simulink model "BaseEngineController_A02_bak"  (Simulink 7.7)
settings: Solver=FixedStepDiscrete  StartTime=0.0  StopTime=10.0  RelTol=1e-3
PreLoadFcn:
  | try, eval([bdroot '_setup']); catch, eval('model_setup', ''); end
InitFcn:
  | try, eval([bdroot '_setup']); catch, eval('model_setup', ''); end
undefined symbols (not defined in the file or in its folder): ECU555, High, motohawk_build_version, motohawk_get_faults, motohawk_trunc_name, motohawk_vardec_config_group, motohawk_vardec_path, True
libraries: MotoHawk_lib x1145, AutoDoc_lib x786, motohawk_control_primitives_lib x114, motohawk_engine_lib x19, motohawk_annotations_lib x12, simulink x11, simulink_extras x1
(part 5 of 23)

== system BaseEngineController_A02_bak/Foreground/Control/Controller/Stall ==
  Enable : EnablePort  StatesWhenEnabling=reset
  Bus Creator2 : BusCreator  Inputs=11
  Closed Throttle : Constant  Value=0
  Documentation_Calibrations_Probes_Block : AutoDoc_lib/Documentation_Calibrations_Probes_Block
  Documentation_Left_Border : AutoDoc_lib/Documentation_Left_Border  layout=Portrait
  Documentation_Right_Border : AutoDoc_lib/Documentation_Right_Border  layout=Portrait
  Documentation_Title_Block : AutoDoc_lib/Documentation_Title_Block
  EGR : Constant  Value=0  OutDataTypeStr=double
  Fuel Pump at Startup : Subsystem
  No Fuel : Constant  Value=0
  No Mult : Constant  Value=0  OutDataTypeStr=double
  No Spark : Constant  Value=0
  No Spark Timing : Constant  Value=0
  No Start EOI : Constant  Value=0
  No Start Fuel Timing : Constant  Value=0
  O2 Heater : Constant  OutDataTypeStr=boolean
  Oil Pump at Startup : Subsystem
  Zero-Order Hold{1..11} (11 alike) : ZeroOrderHold  SampleTime=-1
  motohawk_replicate : MotoHawk_lib/Extra Development Blocks/motohawk_replicate  output_width=-1
  motohawk_replicate2 : MotoHawk_lib/Extra Development Blocks/motohawk_replicate  output_width=-1
  Actuators : Outport
  wires:
  Zero-Order Hold6/1 -> Bus Creator2/1  "FPC"
  No Spark/1 -> Zero-Order Hold4/1
  No Spark Timing/1 -> motohawk_replicate2/1
  No Fuel/1 -> motohawk_replicate/1
  Zero-Order Hold8/1 -> Bus Creator2/2  "SOI"
  No Start Fuel Timing/1 -> Zero-Order Hold8/1
  Bus Creator2/1 -> Actuators/1
  Closed Throttle/1 -> Zero-Order Hold9/1
  Zero-Order Hold4/1 -> Bus Creator2/4  "SparkEnergy"
  Zero-Order Hold3/1 -> Bus Creator2/5  "SparkAdv"
  Zero-Order Hold9/1 -> Bus Creator2/6  "Throttle"
  Zero-Order Hold7/1 -> Bus Creator2/7  "FUELP"
  motohawk_replicate/1 -> Zero-Order Hold6/1
  Zero-Order Hold5/1 -> Bus Creator2/3  "EOI"
  No Start EOI/1 -> Zero-Order Hold5/1
  motohawk_replicate2/1 -> Zero-Order Hold3/1
  No Mult/1 -> Zero-Order Hold10/1
  Zero-Order Hold10/1 -> Bus Creator2/8  "FuelMult"
  Fuel Pump at Startup/1(FUELP [bool]) -> Zero-Order Hold7/1
  EGR/1 -> Zero-Order Hold1/1
  Zero-Order Hold1/1 -> Bus Creator2/9  "EGR"
  Oil Pump at Startup/1(OILP [bool]) -> Zero-Order Hold2/1
  Zero-Order Hold2/1 -> Bus Creator2/10  "OILP"
  O2 Heater/1 -> Zero-Order Hold11/1
  Zero-Order Hold11/1 -> Bus Creator2/11  "O2 Heater"

== system BaseEngineController_A02_bak/Foreground/Control/Diagnostics/Engine Overheat Fault Logic ==
  Sensors : Inport
  Bus Selector : BusSelector  OutputSignals=Raw.NonLinearSensors.ECT
  Documentation_Calibrations_Probes_Block : AutoDoc_lib/Documentation_Calibrations_Probes_Block
  Documentation_Left_Border : AutoDoc_lib/Documentation_Left_Border  layout=Portrait
  Documentation_Right_Border : AutoDoc_lib/Documentation_Right_Border  layout=Portrait
  Documentation_Title_Block : AutoDoc_lib/Documentation_Title_Block
  Logical Operator1 : Logic  Operator=OR  AllPortsSameDT=off  OutDataTypeStr=boolean
  Logical Operator2 : Logic  Inputs=3  AllPortsSameDT=off  OutDataTypeStr=boolean
  Logical Operator3 : Logic  Operator=NOT  Inputs=3  AllPortsSameDT=off  OutDataTypeStr=boolean
  Logical Operator4 : Logic  Inputs=3  AllPortsSameDT=off  OutDataTypeStr=boolean
  Relational Operator1 : RelationalOperator  OutDataTypeStr=boolean
  Relational Operator2 : RelationalOperator  OutDataTypeStr=boolean
  Relational Operator3 : RelationalOperator  OutDataTypeStr=boolean
  Run State : motohawk_control_primitives_lib/CompareTo  SystemSampleTime=-1  op===  val=3
  motohawk_calibration1 : MotoHawk_lib/Calibration & Probing Blocks/motohawk_calibration  nam='TempOverheat_Warn'  val=95  storage=Calibration  show_more=on  name_source=Use Parameter  data_type=double  read_level=1  write_level=1  ovr_cals=on  view_as=Number  enum_struct={'Disabled', 'Enabled'}  vector_style=Wide Row  use_help_units=on  help='ECT limit above which warning light comes on'  units='degC'  use_header_enum=off  row_header_enum={}  col_header_enum={}  use_min_max=off  min_val=-inf  max_val=inf  use_precision=off  precision=''  gain=1  offset=0  exp=1  use_mototune_group=on  mototune_group=motohawk_vardec_path(gcb)
  motohawk_calibration2 : MotoHawk_lib/Calibration & Probing Blocks/motohawk_calibration  nam='TempOverheat_Fault'  val=100  storage=Calibration  show_more=on  name_source=Use Parameter  data_type=double  read_level=1  write_level=1  ovr_cals=on  view_as=Number  enum_struct={'Disabled', 'Enabled'}  vector_style=Wide Row  use_help_units=on  help='ECT limit above which fault is set.'  units='degC'  use_header_enum=off  row_header_enum={}  col_header_enum={}  use_min_max=off  min_val=-inf  max_val=inf  use_precision=off  precision=''  gain=1  offset=0  exp=1  use_mototune_group=on  mototune_group=motohawk_vardec_path(gcb)
  motohawk_calibration3 : MotoHawk_lib/Calibration & Probing Blocks/motohawk_calibration  nam='TempOverheatSevere_Fault'  val=110  storage=Calibration  show_more=on  name_source=Use Parameter  data_type=double  read_level=1  write_level=1  ovr_cals=on  view_as=Number  enum_struct={'Disabled', 'Enabled'}  vector_style=Wide Row  use_help_units=on  help='ECT limit above which severe overheat fault is set.'  units='degC'  use_header_enum=off  row_header_enum={}  col_header_enum={}  use_min_max=off  min_val=-inf  max_val=inf  use_precision=off  precision=''  gain=1  offset=0  exp=1  use_mototune_group=on  mototune_group=motohawk_vardec_path(gcb)
  motohawk_data_read : MotoHawk_lib/Data Storage Blocks/motohawk_data_read  source=Lookup By Name  structure=Scalar  operation=Read entire data structure at once  sz_vec=4  sz_row=2  sz_col=3  typ=uint8  nam='EngineState'  checkNull=on  struct_inst_name='Struct1'  struct_nam='MyStruct'
  motohawk_fault_def1 : MotoHawk_lib/Fault Management Blocks/motohawk_fault_def  nam='ECTOverTempFault'  mode=Enabled  id=[]  x=25  y=50  input_suspected=on  indeterminate=off  increment_xy=on  downsample_count=1  ovr_cals=on  ovr_actions=on  show_actions=off  action1_name=''  action1_cond=(None)  action2_name=''  action2_cond=(None)  action3_name=''  action3_cond=(None)  action4_name=''  action4_cond=(None)
  motohawk_fault_def2 : MotoHawk_lib/Fault Management Blocks/motohawk_fault_def  nam='ECTSevereOverTempFault'  mode=Enabled  id=[]  x=25  y=50  input_suspected=on  indeterminate=off  increment_xy=on  downsample_count=1  ovr_cals=on  ovr_actions=on  show_actions=off  action1_name=''  action1_cond=(None)  action2_name=''  action2_cond=(None)  action3_name=''  action3_cond=(None)  action4_name=''  action4_cond=(None)
  motohawk_fault_status : MotoHawk_lib/Fault Management Blocks/motohawk_fault_status  source=Lookup By Name  nam='ECTRangeHigh'  status=Active
  motohawk_fault_status1 : MotoHawk_lib/Fault Management Blocks/motohawk_fault_status  source=Lookup By Name  nam='ECTRangeLow'  status=Active
  Overheat_Warning : Outport
  wires:
  motohawk_data_read/1 -> Run State/1
  Bus Selector/1 -> Relational Operator3/1, Relational Operator1/1, Relational Operator2/1  "<ECT>"
  motohawk_calibration2/1 -> Relational Operator3/2
  Sensors/1 -> Bus Selector/1
  Relational Operator3/1 -> Logical Operator2/3
  Logical Operator2/1 -> motohawk_fault_def1/1
  Run State/1 -> Logical Operator2/2, Logical Operator4/2
  motohawk_fault_status/1 -> Logical Operator1/1
  motohawk_fault_status1/1 -> Logical Operator1/2
  Logical Operator1/1 -> Logical Operator3/1
  Logical Operator3/1 -> Logical Operator2/1, Logical Operator4/1
  motohawk_calibration1/1 -> Relational Operator1/2
  Relational Operator1/1 -> Overheat_Warning/1
  motohawk_calibration3/1 -> Relational Operator2/2
  Relational Operator2/1 -> Logical Operator4/3
  Logical Operator4/1 -> motohawk_fault_def2/1

== system BaseEngineController_A02_bak/Foreground/Control/Diagnostics/Fault Codes and States ==
  IC : Inport
  Add : Sum  Inputs=+-  OutDataTypeStr=Inherit: Same as first input
  Constant : Constant  Value=uint8(1)
  Constant1 : Constant  Value=length(motohawk_get_faults(bdroot))
  Data Type Conversion : DataTypeConversion  SaturateOnIntegerOverflow=on  RndMeth=Zero
  Data Type Conversion1 : DataTypeConversion  SaturateOnIntegerOverflow=on  RndMeth=Zero
  Documentation_Calibrations_Probes_Block : AutoDoc_lib/Documentation_Calibrations_Probes_Block
  Documentation_Left_Border : AutoDoc_lib/Documentation_Left_Border  layout=Portrait
  Documentation_Right_Border : AutoDoc_lib/Documentation_Right_Border  layout=Portrait
  Documentation_Title_Block : AutoDoc_lib/Documentation_Title_Block
  Relational Operator : RelationalOperator  Operator=<  OutDataTypeStr=boolean
  Terminator : Terminator
  While Iterator : WhileIterator  MaxIters=-1  ResetStates=reset  ShowIterationPort=on  OutputDataType=int8
  motohawk_data_def : MotoHawk_lib/Data Storage Blocks/motohawk_data_def  nam='FltCodes'  container_nam=''  data=zeros(length(motohawk_get_faults(bdroot)),1)  typ=uint16  struct_nam='MyStruct'  storage=Volatile  outputRef=off  use_vardec=off  window=Display  read_level=1  write_level=(Read Only)  ovr_cals=on  view_as=Number  enum_struct={'Disabled', 'Enabled'}  vector_style=Tall Column  use_help_units=off  help=''  units=''  use_header_enum=off  row_header_enum=''  col_header_enum=''  use_min_max=off  min_val=-inf  max_val=inf  use_precision=off  precision=''  gain=1  offset=0  exp=1  use_mototune_group=on  mototune_group='TESTING'  eval_name=FltCodes  data_stores=motohawk_sfun_data_def_data_stores  struct_instances=motohawk_sfun_data_def_struct_instances  vardecs=motohawk_sfun_data_def_vardecs
  motohawk_data_def1 : MotoHawk_lib/Data Storage Blocks/motohawk_data_def  nam='FltActvStatus'  container_nam=''  data=zeros(length(motohawk_get_faults(bdroot)),1)  typ=boolean  struct_nam='MyStruct'  storage=Volatile  outputRef=off  use_vardec=off  window=Display  read_level=1  write_level=(Read Only)  ovr_cals=on  view_as=Number  enum_struct={'Disabled', 'Enabled'}  vector_style=Tall Column  use_help_units=off  help=''  units=''  use_header_enum=off  row_header_enum=''  col_header_enum=''  use_min_max=off  min_val=-inf  max_val=inf  use_precision=off  precision=''  gain=1  offset=0  exp=1  use_mototune_group=on  mototune_group='TESTING'  eval_name=FltActvStatus  data_stores=motohawk_sfun_data_def_data_stores  struct_instances=motohawk_sfun_data_def_struct_instances  vardecs=motohawk_sfun_data_def_vardecs
  motohawk_data_write : MotoHawk_lib/Data Storage Blocks/motohawk_data_write  source=Lookup By Name  structure=Vector  operation=Write scalar into location by index  sz_vec=-1  sz_row=3  sz_col=2  typ=uint16  nam='FltCodes'  checkNull=on  write_read_only=off  struct_inst_name='Struct1'  struct_name='MyStruct'
  motohawk_data_write1 : MotoHawk_lib/Data Storage Blocks/motohawk_data_write  source=Lookup By Name  structure=Vector  operation=Write scalar into location by index  sz_vec=-1  sz_row=3  sz_col=2  typ=boolean  nam='FltActvStatus'  checkNull=on  write_read_only=off  struct_inst_name='Struct1'  struct_name='MyStruct'
  motohawk_fault_iterator : MotoHawk_lib/Fault Management Blocks/motohawk_fault_iterator  criteria=All Faults  loop=off
  motohawk_fault_properties : MotoHawk_lib/Fault Management Blocks/motohawk_fault_properties  source=Input Reference Signal  nam='Fault1'  use_id=on  use_mode=off  use_xlim=off  use_ylim=off  use_x=off  use_y=off
  motohawk_fault_status : MotoHawk_lib/Fault Management Blocks/motohawk_fault_status  source=Input Reference Signal  nam='Fault1'  status=Active
  motohawk_prev_fault_T : MotoHawk_lib/Fault Management Blocks/motohawk_prev_fault_T
  wires:
  Constant/1 -> Add/2
  Data Type Conversion/1 -> Add/1, Relational Operator/1
  Relational Operator/1 -> While Iterator/1
  While Iterator/1 -> Data Type Conversion/1
  Constant1/1 -> Relational Operator/2
  IC/1 -> While Iterator/2
  motohawk_fault_iterator/2 -> motohawk_fault_properties/1, motohawk_prev_fault_T/1, motohawk_fault_status/1
  motohawk_fault_iterator/1 -> Terminator/1
  motohawk_fault_properties/1 -> Data Type Conversion1/1
  Add/1 -> motohawk_data_write/2, motohawk_data_write1/2
  motohawk_fault_status/1 -> motohawk_data_write1/1
  Data Type Conversion1/1 -> motohawk_data_write/1
  motohawk_prev_fault_T/1 -> motohawk_fault_iterator/1

== system BaseEngineController_A02_bak/Foreground/Control/Diagnostics/Freeze Frame Trigger ==
  Active Fault Array : MotoHawk_lib/Fault Management Blocks/motohawk_fault_statuses  nam=motohawk_get_faults(bdroot)  status=Active
  Add : Sum  Inputs=+-  OutDataTypeStr=int8
  Data Type Conversion1 : DataTypeConversion  OutDataTypeStr=boolean  SaturateOnIntegerOverflow=on  RndMeth=Zero
  Documentation_Calibrations_Probes_Block : AutoDoc_lib/Documentation_Calibrations_Probes_Block
  Documentation_Left_Border : AutoDoc_lib/Documentation_Left_Border  layout=Portrait
  Documentation_Right_Border : AutoDoc_lib/Documentation_Right_Border  layout=Portrait
  Documentation_Title_Block : AutoDoc_lib/Documentation_Title_Block
  Freeze-Frame Trigger : Stateflow chart  TreatAsAtomicUnit=on
  Ground1 : Ground
  Initialized at 1 : UnitDelay  X0=1  SampleTime=-1
  Multiport Switch : MultiPortSwitch  DataPortOrder=Zero-based contiguous  Inputs=2  zeroidx=on  InputSameDT=on
  Saturation : Saturate  UpperLimit=1  LowerLimit=0
  Unit Delay2 : UnitDelay  SampleTime=-1
  Width1 : Width  DataType=uint8
  Width2 : Width  DataType=uint8
  motohawk_data_def1 : MotoHawk_lib/Data Storage Blocks/motohawk_data_def  nam='FFIDs'  container_nam=''  data=zeros(6,1)  typ=uint8  struct_nam='MyStruct'  storage=NonVolatile  outputRef=off  use_vardec=off  window=Display  read_level=1  write_level=(Read Only)  ovr_cals=on  view_as=Number  enum_struct={'Disabled', 'Enabled'}  vector_style=Tall Column  use_help_units=off  help=''  units=''  use_header_enum=off  row_header_enum={}  col_header_enum={}  use_min_max=off  min_val=-inf  max_val=inf  use_precision=off  precision=''  gain=1  offset=0  exp=1  use_mototune_group=on  mototune_group='TESTING'  eval_name=FFIDs  data_stores=motohawk_sfun_data_def_data_stores  struct_instances=motohawk_sfun_data_def_struct_instances  vardecs=motohawk_sfun_data_def_vardecs
  motohawk_data_def2 : MotoHawk_lib/Data Storage Blocks/motohawk_data_def  nam='FltOrder'  container_nam=''  data=zeros(length(motohawk_get_faults(bdroot)),1)  typ=uint16  struct_nam='MyStruct'  storage=NonVolatile  outputRef=off  use_vardec=off  window=Display  read_level=1  write_level=(Read Only)  ovr_cals=on  view_as=Number  enum_struct={'Disabled', 'Enabled'}  vector_style=Tall Column  use_help_units=off  help=''  units=''  use_header_enum=off  row_header_enum={}  col_header_enum={}  use_min_max=off  min_val=-inf  max_val=inf  use_precision=off  precision=''  gain=1  offset=0  exp=1  use_mototune_group=on  mototune_group='TESTING'  eval_name=FltOrder  data_stores=motohawk_sfun_data_def_data_stores  struct_instances=motohawk_sfun_data_def_struct_instances  vardecs=motohawk_sfun_data_def_vardecs
  motohawk_data_def3 : MotoHawk_lib/Data Storage Blocks/motohawk_data_def  nam='FltOrderMax'  container_nam=''  data=0  typ=uint16  struct_nam='MyStruct'  storage=NonVolatile  outputRef=off  use_vardec=off  window=Display  read_level=1  write_level=(Read Only)  ovr_cals=on  view_as=Number  enum_struct={'Disabled', 'Enabled'}  vector_style=Tall Column  use_help_units=off  help=''  units=''  use_header_enum=off  row_header_enum={}  col_header_enum={}  use_min_max=off  min_val=-inf  max_val=inf  use_precision=off  precision=''  gain=1  offset=0  exp=1  use_mototune_group=off  mototune_group='TESTING'  eval_name=FltOrderMax  data_stores=motohawk_sfun_data_def_data_stores  struct_instances=motohawk_sfun_data_def_struct_instances  vardecs=motohawk_sfun_data_def_vardecs
  motohawk_data_read1 : MotoHawk_lib/Data Storage Blocks/motohawk_data_read  source=Lookup By Name  structure=Vector  operation=Read entire data structure at once  sz_vec=length(motohawk_get_faults(bdroot))  sz_row=2  sz_col=3  typ=uint16  nam='FltOrder'  checkNull=on  struct_inst_name='Struct1'  struct_nam='MyStruct'
  motohawk_data_read2 : MotoHawk_lib/Data Storage Blocks/motohawk_data_read  source=Lookup By Name  structure=Scalar  operation=Read entire data structure at once  sz_vec=10  sz_row=2  sz_col=3  typ=uint16  nam='FltOrderMax'  checkNull=on  struct_inst_name='Struct1'  struct_nam='MyStruct'
  motohawk_data_read3 : MotoHawk_lib/Data Storage Blocks/motohawk_data_read  source=Lookup By Name  structure=Vector  operation=Read entire data structure at once  sz_vec=6  sz_row=2  sz_col=3  typ=uint8  nam='FFIDs'  checkNull=on  struct_inst_name='Struct1'  struct_nam='MyStruct'
  motohawk_data_write1 : MotoHawk_lib/Data Storage Blocks/motohawk_data_write  source=Lookup By Name  structure=Vector  operation=Write entire data structure at once  sz_vec=6  sz_row=10  sz_col=1  typ=uint8  nam='FFIDs'  checkNull=on  write_read_only=off  struct_inst_name='Struct1'  struct_name='MyStruct'
  motohawk_data_write2 : MotoHawk_lib/Data Storage Blocks/motohawk_data_write  source=Lookup By Name  structure=Vector  operation=Write entire data structure at once  sz_vec=length(motohawk_get_faults(bdroot))  sz_row=10  sz_col=1  typ=uint16  nam='FltOrder'  checkNull=on  write_read_only=off  struct_inst_name='Struct1'  struct_name='MyStruct'
  motohawk_data_write3 : MotoHawk_lib/Data Storage Blocks/motohawk_data_write  source=Lookup By Name  structure=Scalar  operation=Write entire data structure at once  sz_vec=10  sz_row=10  sz_col=1  typ=uint16  nam='FltOrderMax'  checkNull=on  write_read_only=off  struct_inst_name='Struct1'  struct_name='MyStruct'
  zeros vector : Constant  Value=zeros(length(motohawk_get_faults(bdroot)),1)  OutDataTypeStr=int8
  zeros vector1 : Constant  Value=[0 0 0 0 0 0]  OutDataTypeStr=boolean
  IDs : Outport
  Trigger : Outport  Port=2
  wires:
  Unit Delay2/1 -> Add/2
  Add/1 -> Saturation/1
  Saturation/1 -> Multiport Switch/2
  Freeze-Frame Trigger/1(FFIDs) -> motohawk_data_write1/1, IDs/1
  motohawk_data_read3/1 -> Freeze-Frame Trigger/3(FFIDsIn), Width1/1
  Width1/1 -> Freeze-Frame Trigger/4(NumFF)
  Freeze-Frame Trigger/3(FltOrder) -> motohawk_data_write2/1
  motohawk_data_read1/1 -> Freeze-Frame Trigger/5(FltOrderIn), Width2/1
  Freeze-Frame Trigger/4(FltOrderMax) -> motohawk_data_write3/1
  motohawk_data_read2/1 -> Freeze-Frame Trigger/7(FltOrderMaxIn)
  Width2/1 -> Freeze-Frame Trigger/6(NumFlts)
  Freeze-Frame Trigger/2(FFTrigg) -> Trigger/1
  zeros vector/1 -> Multiport Switch/3
  Active Fault Array/1 -> Add/1, Unit Delay2/1
  Multiport Switch/1 -> Freeze-Frame Trigger/1(ActvFlts)
  Initialized at 1/1 -> Data Type Conversion1/1
  Ground1/1 -> Initialized at 1/1
  Data Type Conversion1/1 -> Multiport Switch/1
  zeros vector1/1 -> Freeze-Frame Trigger/2(FFTriggZeros)

== system BaseEngineController_A02_bak/Foreground/Control/Diagnostics/Freeze Frames ==
  Data : Inport
  IDs : Inport  Port=2
  Trigger : Inport  Port=3
  Clear FFIDs and FltOrder : Subsystem  TreatAsAtomicUnit=on
  Demux : Demux  Outputs=6
  Demux1 : Demux  Outputs=6
  Documentation_Calibrations_Probes_Block : AutoDoc_lib/Documentation_Calibrations_Probes_Block
  Documentation_Left_Border : AutoDoc_lib/Documentation_Left_Border  layout=Portrait
  Documentation_Right_Border : AutoDoc_lib/Documentation_Right_Border  layout=Portrait
  Documentation_Title_Block : AutoDoc_lib/Documentation_Title_Block
  Freeze Frame Data Storage : Subsystem  TreatAsAtomicUnit=on  mask{FFnum=1}
      mask prompts: FFnum "Freeze Frame Number"
      mask init:
      | % Color
      | r = 220;
      | g = 211;
      | b = 254;
      | color = strcat('[', num2str(r/255), ',', num2str(g/255), ',', num2str(b/255), ']');
      | motohawk_set_param(gcb, 'BackgroundColor', ...
      |     color);
      | 
      | % Size
      | p = get_param(gcb, 'Position');
      | ht = 52;
      | wd = 290;
      | motohawk_set_param(gcb, 'Position', [p(1) p(2) (p(1) + wd) (p(2) + ht)]);
      | 
      | %Label
      | lbl = ['Freeze-Frame Number: ' num2str(FFnum)];
      mask Display: disp(lbl)
  Freeze Frame Data Storage1 : Subsystem  TreatAsAtomicUnit=on  mask{FFnum=2}
      mask prompts: FFnum "Freeze Frame Number"
      mask init: same as BaseEngineController_A02_bak/Foreground/Control/Diagnostics/Freeze Frames/Freeze Frame Data Storage
      mask Display: disp(lbl)
  Freeze Frame Data Storage2 : Subsystem  TreatAsAtomicUnit=on  mask{FFnum=3}
      mask prompts: FFnum "Freeze Frame Number"
      mask init: same as BaseEngineController_A02_bak/Foreground/Control/Diagnostics/Freeze Frames/Freeze Frame Data Storage
      mask Display: disp(lbl)
  Freeze Frame Data Storage3 : Subsystem  TreatAsAtomicUnit=on  mask{FFnum=4}
      mask prompts: FFnum "Freeze Frame Number"
      mask init: same as BaseEngineController_A02_bak/Foreground/Control/Diagnostics/Freeze Frames/Freeze Frame Data Storage
      mask Display: disp(lbl)
  Freeze Frame Data Storage4 : Subsystem  TreatAsAtomicUnit=on  mask{FFnum=5}
      mask prompts: FFnum "Freeze Frame Number"
      mask init: same as BaseEngineController_A02_bak/Foreground/Control/Diagnostics/Freeze Frames/Freeze Frame Data Storage
      mask Display: disp(lbl)
  Freeze Frame Data Storage5 : Subsystem  TreatAsAtomicUnit=on  mask{FFnum=6}
      mask prompts: FFnum "Freeze Frame Number"
      mask init: same as BaseEngineController_A02_bak/Foreground/Control/Diagnostics/Freeze Frames/Freeze Frame Data Storage
      mask Display: disp(lbl)
  Logical Operator : Logic  Operator=OR  OutDataTypeStr=boolean
  motohawk_calibration : MotoHawk_lib/Calibration & Probing Blocks/motohawk_calibration  nam='ClearFreezeFrames'  val=0  storage=Display  show_more=on  name_source=Use Parameter  data_type=boolean  read_level=1  write_level=1  ovr_cals=on  view_as=Enumeration  enum_struct={'No Clear', 'Clear'}  vector_style=Wide Row  use_help_units=on  help='Manual clearing of freeze-frame data'  units=''  use_header_enum=off  row_header_enum={}  col_header_enum={}  use_min_max=off  min_val=-inf  max_val=inf  use_precision=off  precision=''  gain=1  offset=0  exp=1  use_mototune_group=on  mototune_group=motohawk_vardec_path(gcb)
  motohawk_data_def : MotoHawk_lib/Data Storage Blocks/motohawk_data_def  nam='MyData'  container_nam='FreezeFramesContainer'  data={'FF1', 'FF2','FF3','FF4','FF5','FF6'}  typ=struct container  struct_nam='FreezeFrames'  storage=Volatile  outputRef=off  use_vardec=on  window=Display  read_level=1  write_level=(Read Only)  ovr_cals=on  view_as=Number  enum_struct={'Disabled', 'Enabled'}  vector_style=Wide Row  use_help_units=off  help=''  units=''  use_header_enum=off  row_header_enum=''  col_header_enum=''  use_min_max=off  min_val=-inf  max_val=inf  use_precision=off  precision=''  gain=1  offset=0  exp=1  use_mototune_group=on  mototune_group='Diagnostics & Protection | Faults | Freeze Frame'  eval_name=MyData  data_stores=motohawk_sfun_data_def_data_stores  struct_instances=motohawk_sfun_data_def_struct_instances  vardecs=motohawk_sfun_data_def_vardecs
  motohawk_struct_decl : MotoHawk_lib/Data Storage Blocks/motohawk_struct_decl  struct_nam='FreezeFrames'  data=struct('Name', int32(0), 'Code', int32(0), 'Var1', int32(0), 'Var2', int32(0), 'Var3', int32(0), 'Var4', int32(0), 'Var5', int32(0), 'Var6', int32(0), 'Var7', int32(0), 'Var8', int32(0), 'Var9', int32(0), 'Var10', int32(0))  enum_defs={['None'; motohawk_get_faults(bdroot)]}
  wires:
  Logical Operator/1 -> Demux1/1
  IDs/1 -> Demux/1
  Demux/1 -> Freeze Frame Data Storage/1(FaultID)
  Demux/2 -> Freeze Frame Data Storage1/1(FaultID)
  Demux/3 -> Freeze Frame Data Storage2/1(FaultID)
  Demux/4 -> Freeze Frame Data Storage3/1(FaultID)
  Demux/5 -> Freeze Frame Data Storage4/1(FaultID)
  Demux/6 -> Freeze Frame Data Storage5/1(FaultID)
  Data/1 -> Freeze Frame Data Storage4/2(Data), Freeze Frame Data Storage5/2(Data), Freeze Frame Data Storage3/2(Data), Freeze Frame Data Storage2/2(Data), Freeze Frame Data Storage1/2(Data), Freeze Frame Data Storage/2(Data)
  Demux1/1 -> Freeze Frame Data Storage/Trigger
  Demux1/2 -> Freeze Frame Data Storage1/Trigger
  Demux1/3 -> Freeze Frame Data Storage2/Trigger
  Demux1/4 -> Freeze Frame Data Storage3/Trigger
  Demux1/5 -> Freeze Frame Data Storage4/Trigger
  Demux1/6 -> Freeze Frame Data Storage5/Trigger
  Trigger/1 -> Logical Operator/2
  motohawk_calibration/1 -> Logical Operator/1, Clear FFIDs and FltOrder/Trigger

== system BaseEngineController_A02_bak/Foreground/Control/Diagnostics/Fuel Economy ==
  Sensors : Inport
  Controller : Inport  Port=2
  Bus Selector : BusSelector  OutputSignals=Raw.CAN Inputs.112.Vehicle Speed
  Bus Selector1 : BusSelector  OutputSignals=FPC
  Bus Selector2 : BusSelector  OutputSignals=Raw.RPM
  Bus Selector3 : BusSelector  OutputSignals=Raw.LinearSensors.FuelSense
  Calculate Fuel Density : Subsystem
  Constant1 : Constant
  Constant2 : Constant
  Data Type Conversion : DataTypeConversion  SaturateOnIntegerOverflow=on  RndMeth=Zero
  Default (if no flow) : Switch  Threshold=0.5  ZeroCross=off  SaturateOnIntegerOverflow=on  InputSameDT=on
  Default value : Constant  Value=0
  Documentation_Calibrations_Probes_Block : AutoDoc_lib/Documentation_Calibrations_Probes_Block
  Documentation_Left_Border : AutoDoc_lib/Documentation_Left_Border  layout=Portrait
  Documentation_Right_Border : AutoDoc_lib/Documentation_Right_Border  layout=Portrait
  Documentation_Title_Block : AutoDoc_lib/Documentation_Title_Block
  First Order Low Pass (Tunable) : Subsystem  AncestorBlock=motohawk_control_primitives_lib/First Order Low Pass (Tunable)  mask(First-Order Low-Pass Filter (Tunable)){nam='FuelEconomy_Filter', samp_time=-1, group=motohawk_vardec_path(gcb)}
      mask prompts: nam "Name"; samp_time "Sample Time"; group "MotoTune Group String"
      mask help:
      | Discrete first-order low-pass filter, with calibratable time-constant.
      |
      | If 'Sample Time' is -1, then extra code and RAM will be generated to dynamically calculate dt.
      mask Display:
      | patch([0 0 1 1], [0 0.075 0.075 0], [230/255 0/255 0/255])
      | patch([0 0 1 1], [0.025 0.05 0.05 0.025], [150/255 0/255 0/255])
      | patch([0 0 1 1], [0 0.025 0.025 0], [0/255 0/255 0/255])
      | 
      | disp('1st-Order\nLow Pass');
  Mass flow to Volume flow mg/hr to ml/hr : Product  Inputs=*/  SaturateOnIntegerOverflow=on  InputSameDT=on  OutDataTypeStr=Inherit: Same as first input
  Min fuel flow  for calc : Constant  Value=0.1
  "Multiply and Divide, avoiding divde by zero1" : motohawk_engine_lib/Mass Flow to Charge Mass Transform/Multiply and Divide, avoiding divde by zero  SystemSampleTime=-1
  Number of Combustion Events Per Revolution : MotoHawk_lib/Data Storage Blocks/motohawk_data_read  source=Lookup By Name  structure=Scalar  operation=Read scalar from location by index  sz_vec=-1  sz_row=-1  sz_col=-1  typ=uint8  nam='CombEventsPerRev'  checkNull=on  struct_inst_name='Struct1'  struct_nam='MyStruct'
  RPM to inj/min : Product  RndMeth=Zero
  Relational Operator7 : RelationalOperator  Operator=>  OutDataTypeStr=boolean
  g to mg : Gain  Gain=1000
  inj/hr to mg/hr : Product  RndMeth=Zero
  inj/min to inj/hr : Gain  Gain=60
  ml/hr to gal/hr : Gain  Gain=1/3785.41178
  FuelEconomy_instantaneous : Outport
  FuelEconomy_filtered : Outport  Port=2
  wires:
  Sensors/1 -> Bus Selector/1, Bus Selector2/1, Bus Selector3/1
  Controller/1 -> Bus Selector1/1
  Bus Selector2/1 -> RPM to inj/min/1  "<RPM>"
  Number of Combustion Events Per Revolution/1 -> Data Type Conversion/1
  RPM to inj/min/1 -> inj/min to inj/hr/1
  inj/min to inj/hr/1 -> inj/hr to mg/hr/1
  Bus Selector1/1 -> inj/hr to mg/hr/2  "<FPC>"
  Bus Selector3/1 -> Calculate Fuel Density/1(Ethanol_xx [%])  "<FuelSense>"
  Calculate Fuel Density/1(Fuel Density (g/ml)) -> g to mg/1
  Data Type Conversion/1 -> RPM to inj/min/2
  inj/hr to mg/hr/1 -> Mass flow to Volume flow mg/hr to ml/hr/1
  g to mg/1 -> Mass flow to Volume flow mg/hr to ml/hr/2
  Mass flow to Volume flow mg/hr to ml/hr/1 -> ml/hr to gal/hr/1
  Constant2/1 -> "Multiply and Divide, avoiding divde by zero1"/2
  Constant1/1 -> "Multiply and Divide, avoiding divde by zero1"/4
  Bus Selector/1 -> "Multiply and Divide, avoiding divde by zero1"/1  "<Vehicle Speed>"
  ml/hr to gal/hr/1 -> "Multiply and Divide, avoiding divde by zero1"/3, Relational Operator7/1
  Min fuel flow  for calc/1 -> Relational Operator7/2
  Relational Operator7/1 -> "Multiply and Divide, avoiding divde by zero1"/Enable, Default (if no flow)/2
  "Multiply and Divide, avoiding divde by zero1"/1 -> Default (if no flow)/1
  Default value/1 -> Default (if no flow)/3
  Default (if no flow)/1 -> FuelEconomy_instantaneous/1, First Order Low Pass (Tunable)/1(in)
  First Order Low Pass (Tunable)/1(out) -> FuelEconomy_filtered/1

== system BaseEngineController_A02_bak/Foreground/Control/Diagnostics/Low Oil Pressure Fault Logic ==
  Sensors : Inport
  Bus Selector : BusSelector  OutputSignals=RawProcessed.EngineState
  Bus Selector1 : BusSelector  OutputSignals=RawIndexes.RPM09Idx
  Bus Selector4 : BusSelector  OutputSignals=Raw.LinearSensors.OilPres
  Documentation_Calibrations_Probes_Block : AutoDoc_lib/Documentation_Calibrations_Probes_Block
  Documentation_Left_Border : AutoDoc_lib/Documentation_Left_Border  layout=Portrait
  Documentation_Right_Border : AutoDoc_lib/Documentation_Right_Border  layout=Portrait
  Documentation_Title_Block : AutoDoc_lib/Documentation_Title_Block
  Logical Operator1 : Logic  Operator=OR  AllPortsSameDT=off  OutDataTypeStr=boolean
  Logical Operator3 : Logic  Operator=NOT  Inputs=3  AllPortsSameDT=off  OutDataTypeStr=boolean
  Logical Operator4 : Logic  Inputs=3  AllPortsSameDT=off  OutDataTypeStr=boolean
  Relational Operator1 : RelationalOperator  Operator=<  OutDataTypeStr=boolean
  Relational Operator2 : RelationalOperator  Operator=<  OutDataTypeStr=boolean
  Relational Operator5 : RelationalOperator  Operator=>  OutDataTypeStr=boolean
  Run State : motohawk_control_primitives_lib/CompareTo  SystemSampleTime=-1  op===  val=3
  Time Since Enabled (With Input)2 : motohawk_control_primitives_lib/Time Since Enabled (With Input)1  SystemSampleTime=-1  nam='OilPresFaultTimer'  samp_time=-1  init=0  upperlim=16000
  motohawk_calibration1 : MotoHawk_lib/Calibration & Probing Blocks/motohawk_calibration  nam='NoOilPres_FaultTime'  val=1  storage=Calibration  show_more=on  name_source=Use Parameter  data_type=double  read_level=1  write_level=1  ovr_cals=on  view_as=Number  enum_struct={'Disabled', 'Enabled'}  vector_style=Wide Row  use_help_units=on  help='After no oil pressure for this long the NoOilFault becomes active.'  units='s'  use_header_enum=off  row_header_enum={}  col_header_enum={}  use_min_max=off  min_val=0  max_val=inf  use_precision=off  precision=''  gain=1  offset=0  exp=1  use_mototune_group=on  mototune_group=motohawk_vardec_path(gcb)
  motohawk_fault_def1 : MotoHawk_lib/Fault Management Blocks/motohawk_fault_def  nam='NoOil_Fault'  mode=Sticky  id=[]  x=1  y=2  input_suspected=on  indeterminate=off  increment_xy=on  downsample_count=1  ovr_cals=on  ovr_actions=on  show_actions=on  action1_name='EngineShutdown'  action1_cond=Active  action2_name=''  action2_cond=(None)  action3_name=''  action3_cond=(None)  action4_name=''  action4_cond=(None)
  motohawk_fault_status : MotoHawk_lib/Fault Management Blocks/motohawk_fault_status  source=Lookup By Name  nam='OilPresRangeHigh'  status=Active
  motohawk_fault_status1 : MotoHawk_lib/Fault Management Blocks/motohawk_fault_status  source=Lookup By Name  nam='OilPresRangeLow'  status=Active
  motohawk_interpolation_1d : MotoHawk_lib/Lookup Tables/motohawk_interpolation_1d  nam='MinOilPressure_Fault'  table_data=[0 10 20 20 30 40 40 50 50]  show_more=on  data_type=Inherit from 'Table Data'  storage=NonAdaptive  read_level=1  write_level=1  ovr_cals=on  use_help=on  help='Minimum oil pressure based on RPM - engine faults and shuts down below this'  units='psi'  use_min_max=off  min_val=-inf  max_val=inf  use_precision=off  precision=''  gain=1  offset=0  exp=1  use_links=off  prelookup_block=''  output_name=''  use_mototune_group=on  mototune_group=motohawk_vardec_path(gcb)
  motohawk_interpolation_1d3 : MotoHawk_lib/Lookup Tables/motohawk_interpolation_1d  nam='MinOilPressure_Warn'  table_data=[20 20 20 20 30 30 40 40 50]  show_more=on  data_type=Inherit from 'Table Data'  storage=NonAdaptive  read_level=1  write_level=1  ovr_cals=on  use_help=on  help='Minimum oil pressure based on RPM - indicator light comes on below this'  units='psi'  use_min_max=off  min_val=-inf  max_val=inf  use_precision=off  precision=''  gain=1  offset=0  exp=1  use_links=off  prelookup_block=''  output_name=''  use_mototune_group=on  mototune_group=motohawk_vardec_path(gcb)
  LowOilPres : Outport
  wires:
  Logical Operator4/1 -> Time Since Enabled (With Input)2/1
  Relational Operator5/1 -> motohawk_fault_def1/1
  motohawk_calibration1/1 -> Relational Operator5/2
  Time Since Enabled (With Input)2/1 -> Relational Operator5/1
  Sensors/1 -> Bus Selector/1, Bus Selector4/1, Bus Selector1/1
  Logical Operator3/1 -> Logical Operator4/1
  Logical Operator1/1 -> Logical Operator3/1
  motohawk_fault_status1/1 -> Logical Operator1/2
  motohawk_fault_status/1 -> Logical Operator1/1
  Bus Selector/1 -> Run State/1  "<EngineState>"
  Relational Operator1/1 -> LowOilPres/1
  motohawk_interpolation_1d3/1 -> Relational Operator1/2
  Bus Selector1/1 -> motohawk_interpolation_1d/1, motohawk_interpolation_1d3/1  "<RPM09Idx>"
  motohawk_interpolation_1d/1 -> Relational Operator2/2
  Bus Selector4/1 -> Relational Operator2/1, Relational Operator1/1  "<OilPres>"
  Run State/1 -> Logical Operator4/2
  Relational Operator2/1 -> Logical Operator4/3

== system BaseEngineController_A02_bak/Foreground/Control/Diagnostics/Low System Voltage Fault Logic ==
  Sensors : Inport
  Bus Selector : BusSelector  OutputSignals=RawProcessed.EngineState
  Bus Selector4 : BusSelector  OutputSignals=Raw.LinearSensors.SysVolt
  Documentation_Calibrations_Probes_Block : AutoDoc_lib/Documentation_Calibrations_Probes_Block
  Documentation_Left_Border : AutoDoc_lib/Documentation_Left_Border  layout=Portrait
  Documentation_Right_Border : AutoDoc_lib/Documentation_Right_Border  layout=Portrait
  Documentation_Title_Block : AutoDoc_lib/Documentation_Title_Block
  Logical Operator1 : Logic  Operator=OR  AllPortsSameDT=off  OutDataTypeStr=boolean
  Logical Operator3 : Logic  Operator=NOT  Inputs=3  AllPortsSameDT=off  OutDataTypeStr=boolean
  Logical Operator4 : Logic  Inputs=3  AllPortsSameDT=off  OutDataTypeStr=boolean
  Relational Operator2 : RelationalOperator  Operator=<  OutDataTypeStr=boolean
  Relational Operator5 : RelationalOperator  Operator=>  OutDataTypeStr=boolean
  Run State : motohawk_control_primitives_lib/CompareTo  SystemSampleTime=-1  op===  val=3
  Time Since Enabled (With Input)2 : motohawk_control_primitives_lib/Time Since Enabled (With Input)1  SystemSampleTime=-1  nam='SysVoltLow_FaultTimer'  samp_time=-1  init=0  upperlim=16000
  motohawk_calibration1 : MotoHawk_lib/Calibration & Probing Blocks/motohawk_calibration  nam='SysVolt_Low_FaultTime'  val=5  storage=Calibration  show_more=on  name_source=Use Parameter  data_type=double  read_level=1  write_level=1  ovr_cals=on  view_as=Number  enum_struct={'Disabled', 'Enabled'}  vector_style=Wide Row  use_help_units=on  help='Number of seconds (in run state) that sysvolt must be below thresholdd for fault'  units='s'  use_header_enum=off  row_header_enum={}  col_header_enum={}  use_min_max=off  min_val=0  max_val=inf  use_precision=off  precision=''  gain=1  offset=0  exp=1  use_mototune_group=on  mototune_group=motohawk_vardec_path(gcb)
  motohawk_calibration2 : MotoHawk_lib/Calibration & Probing Blocks/motohawk_calibration  nam='SysVolt_Low_Fault'  val=13.069  storage=Calibration  show_more=on  name_source=Use Parameter  data_type=double  read_level=1  write_level=1  ovr_cals=on  view_as=Number  enum_struct={'Disabled', 'Enabled'}  vector_style=Wide Row  use_help_units=on  help='System voltage below which a charging system fault is set'  units='V'  use_header_enum=off  row_header_enum={}  col_header_enum={}  use_min_max=off  min_val=-inf  max_val=inf  use_precision=off  precision=''  gain=1  offset=0  exp=1  use_mototune_group=on  mototune_group=motohawk_vardec_path(gcb)
  motohawk_fault_def1 : MotoHawk_lib/Fault Management Blocks/motohawk_fault_def  nam='SysVoltLow_Fault'  mode=Enabled  id=[]  x=25  y=50  input_suspected=on  indeterminate=off  increment_xy=on  downsample_count=1  ovr_cals=on  ovr_actions=on  show_actions=on  action1_name=''  action1_cond=(None)  action2_name=''  action2_cond=(None)  action3_name=''  action3_cond=(None)  action4_name=''  action4_cond=(None)
  motohawk_fault_status : MotoHawk_lib/Fault Management Blocks/motohawk_fault_status  source=Lookup By Name  nam='SysVoltRangeHigh'  status=Active
  motohawk_fault_status1 : MotoHawk_lib/Fault Management Blocks/motohawk_fault_status  source=Lookup By Name  nam='SysVoltRangeLow'  status=Active
  wires:
  Logical Operator4/1 -> Time Since Enabled (With Input)2/1
  Relational Operator5/1 -> motohawk_fault_def1/1
  motohawk_calibration1/1 -> Relational Operator5/2
  Time Since Enabled (With Input)2/1 -> Relational Operator5/1
  Sensors/1 -> Bus Selector4/1, Bus Selector/1
  Logical Operator3/1 -> Logical Operator4/1
  Logical Operator1/1 -> Logical Operator3/1
  motohawk_fault_status1/1 -> Logical Operator1/2
  motohawk_fault_status/1 -> Logical Operator1/1
  Bus Selector/1 -> Run State/1  "<EngineState>"
  Bus Selector4/1 -> Relational Operator2/1  "<SysVolt>"
  Run State/1 -> Logical Operator4/2
  Relational Operator2/1 -> Logical Operator4/3
  motohawk_calibration2/1 -> Relational Operator2/2
